simulink model "MCVT_Integration"  (R2020a)
settings: Solver=ode8  StartTime=0.0  StopTime=T_end  FixedStep=dt  RelTol=1e-3  EnableMultiTasking=on
values from neighbouring files (symbols this model uses):
  assumed_power_voltage = 1.25e2    (= 125)    [MCVT_Input_File.m]    (also in MCVT_Run_QuickCheck.m)
  battery_power_toggle = 1    [MCVT_Input_File.m]    (also in MCVT_Run_QuickCheck.m)
  battery_trickle_charge_param = 0.1    [MCVT_Input_File.m]    (also in MCVT_Run_QuickCheck.m)
  battery_trickle_leakage_param = 0.01    [MCVT_Input_File.m]    (also in MCVT_Run_QuickCheck.m)
  damage_case_id = 3    [MCVT_Input_File.m]    (also in MCVT_Run_QuickCheck.m = 1)
  dt = 1/fs    (= 0.001)    [MCVT_Run_QuickCheck.m]    (also in MCVT_Sim_Set.m)
  dt_2 = 1/fs_2    (= 0.001)    [MCVT_Run_QuickCheck.m]    (also in MCVT_Sim_Set.m)
  dt_3 = 1/fs_3    (= 0.001)    [MCVT_Run_QuickCheck.m]    (also in MCVT_Sim_Set.m)
  dt_5 = 1/fs_5    (= 0.005)    [MCVT_Run_QuickCheck.m]    (also in MCVT_Sim_Set.m)
  dt_8 = 1/fs_8    (= 0.05)    [MCVT_Run_QuickCheck.m]    (also in MCVT_Sim_Set.m)
  dt_9 = 1/fs_9    (= 0.001)    [MCVT_Run_QuickCheck.m]    (also in MCVT_Sim_Set.m)
  dust_met_duration = 3    [MCVT_Input_File.m]    (also in MCVT_Run_QuickCheck.m = 2)
  ECLSS_indoor_set_temperature = 298.15    [MCVT_Input_File.m]    (also in MCVT_Run_QuickCheck.m)
  energy_store_max_o = 150    [MCVT_Input_File.m]    (also in MCVT_Run_QuickCheck.m)
  energy_store_o = 120    [MCVT_Input_File.m]    (also in MCVT_Run_QuickCheck.m)
  fs = 1000    [MCVT_Run_QuickCheck.m]    (also in MCVT_Sim_Set.m)
  gain_charge_param = 1    [MCVT_Input_File.m]    (also in MCVT_Run_QuickCheck.m)
  ground_temperature = 373    [MCVT_Habitat_Design_Input_File.m]    (also in MCVT_Run_QuickCheck.m)
  I_max_charge = 800    [MCVT_Input_File.m]    (also in MCVT_Run_QuickCheck.m)
  I_max_discharge = 800    [MCVT_Input_File.m]    (also in MCVT_Run_QuickCheck.m)
  initial_pressure = 1e5    (= 100000)    [MCVT_Input_File.m]    (also in MCVT_Run_QuickCheck.m)
  initial_temperature = 295    [MCVT_Input_File.m]    (also in MCVT_Run_QuickCheck.m)
  interior_leak_rate = 0.00415    [MCVT_Input_File.m]    (also in MCVT_Run_QuickCheck.m)
  launch_dust_accum_start_time = 10    [MCVT_Input_File.m]    (also in MCVT_Run_QuickCheck.m = 2)
  launch_dust_accum_stop_time = 12    [MCVT_Input_File.m]    (also in MCVT_Run_QuickCheck.m = 3)
  meteorite_impact_time = 2    [MCVT_Input_File.m]    (also in MCVT_Run_QuickCheck.m = 1)
  nuclear_power_toggle = 1    [MCVT_Input_File.m]    (also in MCVT_Run_QuickCheck.m)
  print_interval = 30    [MCVT_Input_File.m]
  SOC_lower_thres = 0.5    [MCVT_Input_File.m]    (also in MCVT_Run_QuickCheck.m)
  SOC_upper_thres = 0.9    [MCVT_Input_File.m]    (also in MCVT_Run_QuickCheck.m)
  solar_power_toggle = 1    [MCVT_Input_File.m]    (also in MCVT_Run_QuickCheck.m)
  space_temperature = 4    [MCVT_Habitat_Design_Input_File.m]    (also in MCVT_Run_QuickCheck.m)
  Start_angle = 90    [MCVT_Input_File.m]    (also in MCVT_Run_QuickCheck.m)
undefined symbols (not defined in the file or in its folder): A_flow_restrictor, ACTIVITY_LOOKUP_TABLE, air, Aref, Bmap, Bmap2, Bmap3, comms, DiscIntNeverNeededParam, DmgSPL, dust_parameters, ECLSS_dust_damage_repair_time, ECLSS_heater_capacity, ECLSS_IPS_restrictor, ECLSS_IPS_setpointPressure, ECLSS_paint_damage_repair_time, ECLSS_radiatorLoop_discreteNumber, ECLSS_Vst, emptyActivity, expected_heat_add, FI, habitat, HIEM2SMM, HIEM2STM, HIEMDamageElements, impact_numbering, initial_SPLT_damage_index, input_air, input_Cond, input_CP, input_discretization, input_EV, input_Evap, input_radiatorLoop, K_ECLSS_dust_single, K_ECLSS_paint_single, K_Structure_damage_single, Kref, kW_to_W, meteoroidDustDep, mFRF, ndelays, nominalDustDep, noverlap, nuclear_toggle_gain, output_limits, panel_paint0, Ps0, reference_temperature, RepairTime_ECLSS_factor, Sensor_Location_SMM, SMM_T0, SMM_T1, SMM_T2, SMM_T3, SMM_T4, SMM_T4_0, SMM_T4_1, SMM_T4_2, solar_FDD_K ... (+73)
libraries: neural x350, simulink x39, xpcutilitieslib x7, xpcrtudplib x7, dspstat3 x1
(part 23 of 43)

== system MCVT_Integration/Sys2 - Structural System/Sys2_Structural_System/Fault Detection and Diagnosis (FDD) with Binary Classifer/Pattern Recognition Neural Network/Segment 1/Layer 2/Delays 1 ==
  "p{2}" : Inport  PortDimensions=20
      mask Display: e = 0.06;c1 = [120 230 180]/255;c2 = ([120 230 180]+0)/(256+128);c3 = ([120 230 180]+128)/(256+128);patch([0 1 1 0],[0 0 1 1],c1);patch([0 1 1 1-e 1-e 0+e],[0 0 1 1-e 0+e 0+e],c2);patch([0 0 1 1-e 0+e 0+e],[0 1 1 1-e 1-e 0+e],c3);plot([0 1 1 0 0],[0 0 1 1 0]);
  mux : Mux  Inputs=1
      mask Display: e = 0.06;c1 = [120 150 230]/255;c2 = ([120 150 230]+0)/383;c3 = ([120 150 230]+128)/383;patch([0 1 1 0],[0 0 1 1],c1);patch([0 1 1 1-e 1-e 0+e],[0 0 1 1-e 0+e 0+e],c2);patch([0 0 1 1-e 0+e 0+e],[0 1 1 1-e 1-e 0+e],c3);plot([0 1 1 0 0],[0 0 1 1 0]);disp('Mux')
  "pd{2,1}" : Outport
      mask Display: e = 0.06;c1 = [120 230 180]/255;c2 = ([120 230 180]+0)/(256+128);c3 = ([120 230 180]+128)/(256+128);patch([0 1 1 0],[0 0 1 1],c1);patch([0 1 1 1-e 1-e 0+e],[0 0 1 1-e 0+e 0+e],c2);patch([0 0 1 1-e 0+e 0+e],[0 1 1 1-e 1-e 0+e],c3);plot([0 1 1 0 0],[0 0 1 1 0]);
  wires:
  "p{2}"/1 -> mux/1
  mux/1 -> "pd{2,1}"/1

== system MCVT_Integration/Sys2 - Structural System/Sys2_Structural_System/Fault Detection and Diagnosis (FDD) with Binary Classifer/Pattern Recognition Neural Network/Segment 1/Layer 2/LW{2,1} ==
  "ad{2,1}" : Inport  PortDimensions=20
      mask Display: e = 0.06;c1 = [120 230 180]/255;c2 = ([120 230 180]+0)/(256+128);c3 = ([120 230 180]+128)/(256+128);patch([0 1 1 0],[0 0 1 1],c1);patch([0 1 1 1-e 1-e 0+e],[0 0 1 1-e 0+e 0+e],c2);patch([0 0 1 1-e 0+e 0+e],[0 1 1 1-e 1-e 0+e],c3);plot([0 1 1 0 0],[0 0 1 1 0]);
  "IW{2,1}(1,:)'" : Constant  Value=[-0.25104247195081141885708575500757433474063873291015625;-0.07087667517882868806200491462732315994799137115478515625;-0.50138857313681939320559877160121686756610870361328125;0.470590818650424458358116908129886724054813385009765625;0.10216708641673168111996261586682521738111972808837890625;-0.10534378290408989709003861889868858270347118377685546875;-0.220423474240058869799696594782290048897266387939453125;0.0047890827843588834600296877397340722382068634033203125;-0.172162427952498420236082665724097751080989837646484375;0.02593303469759399015881484729106887243688106536865234375;-0.440084899709885124519104238061117939651012420654296875;0.5753831393220114609476922851172275841236114501953125;0.52466696856238626001101010842830874025821685791015625;-0.027075079370393294320162880239877267740666866302490234375;0.2061943639996793586899315187110914848744869232177734375;-0.65033892131392878610540719819255173206329345703125;-0.54391815389803876978902508199098519980907440185546875;-0.136603011825610132223829396025394089519977569580078125;-0.4909008044092624256649060043855570256710052490234375;-0.43293585150828750585105808568187057971954345703125]
      mask Display: e = 0.06;c1 = [120 150 230]/255;c2 = ([120 150 230]+0)/383;c3 = ([120 150 230]+128)/383;patch([0 1 1 0],[0 0 1 1],c1);patch([0 1 1 1-e 1-e 0+e],[0 0 1 1-e 0+e 0+e],c2);patch([0 0 1 1-e 0+e 0+e],[0 1 1 1-e 1-e 0+e],c3);plot([0 1 1 0 0],[0 0 1 1 0]);disp('weights');
  "IW{2,1}(10,:)'" : Constant  Value=[-0.0014451714761772004036455285813644877634942531585693359375;0.04608926107551207518309155375391128472983837127685546875;-0.11821960628676408955328724914579652249813079833984375;-0.682229667481613955004604576970450580120086669921875;-0.0159400991711729327071633832701991195790469646453857421875;-0.86523788555619007833996647605090402066707611083984375;-0.003532397103530628135192870331593439914286136627197265625;-0.6176597636085656706228519396972842514514923095703125;0.49329025270850446194259575349860824644565582275390625;-0.5412568517979365889658538435469381511211395263671875;0.473563214439475854700134505037567578256130218505859375;-0.1767114189148812075647043684512027539312839508056640625;-0.399614154169822433093628433198318816721439361572265625;0.07107937000623387735487312966142781078815460205078125;-0.345436571249109036774171954675693996250629425048828125;-0.51026032312097269194595128283253870904445648193359375;0.4013803605705799082414841905119828879833221435546875;0.0283768978295916400600429341238850611262023448944091796875;0.384419257535470293429824550912599079310894012451171875;0.156445817512429863338496716096415184438228607177734375]
      mask Display: e = 0.06;c1 = [120 150 230]/255;c2 = ([120 150 230]+0)/383;c3 = ([120 150 230]+128)/383;patch([0 1 1 0],[0 0 1 1],c1);patch([0 1 1 1-e 1-e 0+e],[0 0 1 1-e 0+e 0+e],c2);patch([0 0 1 1-e 0+e 0+e],[0 1 1 1-e 1-e 0+e],c3);plot([0 1 1 0 0],[0 0 1 1 0]);disp('weights');
  "IW{2,1}(11,:)'" : Constant  Value=[0.42081956059319758356451757208560593426227569580078125;-0.022623835269686531912469718008651398122310638427734375;-0.1912529964857672959421819314229651354253292083740234375;0.411268215249822599588469529408030211925506591796875;-0.03376416723225823812715162830500048585236072540283203125;0.028509914893441935801465803024257184006273746490478515625;0.493459475451891893538203248681384138762950897216796875;-0.2255309221203130876087783462935476563870906829833984375;-0.50410452447975073742014728850335814058780670166015625;0.187049216999234102498661513891420327126979827880859375;0.032548129774071281328229332530099782161414623260498046875;-0.1551786688610629905138438289213809184730052947998046875;0.513329365290914996222682020743377506732940673828125;0.55303888864058825536318408921943046152591705322265625;0.45722816612853822704209960647858679294586181640625;-0.0831223966265599301106448137943516485393047332763671875;-0.35699709311150495949505057069472968578338623046875;-0.50985596418355594305893419004860334098339080810546875;-0.488125509118289946375313093085424043238162994384765625;-0.55527675340083815225256103076389990746974945068359375]
      mask Display: e = 0.06;c1 = [120 150 230]/255;c2 = ([120 150 230]+0)/383;c3 = ([120 150 230]+128)/383;patch([0 1 1 0],[0 0 1 1],c1);patch([0 1 1 1-e 1-e 0+e],[0 0 1 1-e 0+e 0+e],c2);patch([0 0 1 1-e 0+e 0+e],[0 1 1 1-e 1-e 0+e],c3);plot([0 1 1 0 0],[0 0 1 1 0]);disp('weights');
  "IW{2,1}(12,:)'" : Constant  Value=[0.236430354655128016627685383355128578841686248779296875;0.321247634226019596059842342583579011261463165283203125;-0.381619419658791370064676584661356173455715179443359375;-0.5603837391530195333189112716354429721832275390625;-0.34218091312457066255348081540432758629322052001953125;0.250109754436278475253629949293099343776702880859375;0.38122207807599906015383339763502590358257293701171875;-0.11789201686204854591100144034498953260481357574462890625;-0.338756734050894514620466679843957535922527313232421875;0.412391661445961343890331818329286761581897735595703125;0.414075134801581867804998182691633701324462890625;0.038104094557145896937644380386700504459440708160400390625;0.0302419039804045948482968952930605155415832996368408203125;0.4887310275038261320190713377087377011775970458984375;0.1411505209161965368469537907003541477024555206298828125;-0.6258221129402887772386065989849157631397247314453125;0.467298676415856484300803685982828028500080108642578125;-0.42901209579906207647326255028019659221172332763671875;-0.240050243971256904718103442064602859318256378173828125;-0.285545664296158741013442750045214779675006866455078125]
      mask Display: e = 0.06;c1 = [120 150 230]/255;c2 = ([120 150 230]+0)/383;c3 = ([120 150 230]+128)/383;patch([0 1 1 0],[0 0 1 1],c1);patch([0 1 1 1-e 1-e 0+e],[0 0 1 1-e 0+e 0+e],c2);patch([0 0 1 1-e 0+e 0+e],[0 1 1 1-e 1-e 0+e],c3);plot([0 1 1 0 0],[0 0 1 1 0]);disp('weights');
  "IW{2,1}(13,:)'" : Constant  Value=[-0.70188226939873032961259013973176479339599609375;-0.052550375883878598648468738474548445083200931549072265625;-0.2149029163891983673639884955264278687536716461181640625;0.4523966484412869082376573715009726583957672119140625;0.447030058856958290025573887760401703417301177978515625;0.91880597605265312832756308125681243836879730224609375;0.332584103805542985465848460080451332032680511474609375;0.474859162877379503697738982737064361572265625;-0.78858829848239386794972460847930051386356353759765625;-0.250963571223336401327941302952240221202373504638671875;-0.09702683642612723591280854407159495167434215545654296875;0.2178231302834403304569121928579988889396190643310546875;-0.0090886058850562327637856441242547589354217052459716796875;-0.053394177694803111877686063735382049344480037689208984375;-0.26261695092550108032725120210670866072177886962890625;0.5229767299097236588067971752025187015533447265625;0.433527928048706379104970665139262564480304718017578125;0.6401460177374802551497623426257632672786712646484375;-0.32168396407409549642153478998807258903980255126953125;0.552601658021480535154523749952204525470733642578125]
      mask Display: e = 0.06;c1 = [120 150 230]/255;c2 = ([120 150 230]+0)/383;c3 = ([120 150 230]+128)/383;patch([0 1 1 0],[0 0 1 1],c1);patch([0 1 1 1-e 1-e 0+e],[0 0 1 1-e 0+e 0+e],c2);patch([0 0 1 1-e 0+e 0+e],[0 1 1 1-e 1-e 0+e],c3);plot([0 1 1 0 0],[0 0 1 1 0]);disp('weights');
  "IW{2,1}(14,:)'" : Constant  Value=[0.55502051895556980820600756487692706286907196044921875;0.322359101059607577877130779597791843116283416748046875;0.419005824050040553974127988112741149961948394775390625;0.007681609285077460602675358813939965330064296722412109375;-0.2410459656664609429110868177303927950561046600341796875;-0.221039781054034156770882191267446614801883697509765625;0.54603930521161980760069809548440389335155487060546875;0.067418959358650554225533824137528426945209503173828125;-0.0323460782665744017716491498504183255136013031005859375;-0.1856337082362842227922783422400243580341339111328125;-0.53405205180679649057395863565034233033657073974609375;0.343953276170277655321427801027311943471431732177734375;-0.16456428638465825375902795713045634329319000244140625;0.393861106754853473876920588736538775265216827392578125;-0.4502608918815782512723444597213529050350189208984375;-0.288216798376219440758205792008084245026111602783203125;0.586902267887376094535056836321018636226654052734375;-0.4647318227858097117177749169059097766876220703125;0.346225802719296649190283687858027406036853790283203125;-0.314419441092286866545890688939834944903850555419921875]
      mask Display: e = 0.06;c1 = [120 150 230]/255;c2 = ([120 150 230]+0)/383;c3 = ([120 150 230]+128)/383;patch([0 1 1 0],[0 0 1 1],c1);patch([0 1 1 1-e 1-e 0+e],[0 0 1 1-e 0+e 0+e],c2);patch([0 0 1 1-e 0+e 0+e],[0 1 1 1-e 1-e 0+e],c3);plot([0 1 1 0 0],[0 0 1 1 0]);disp('weights');
  "IW{2,1}(15,:)'" : Constant  Value=[0.261592048245355812685630780833889730274677276611328125;0.258266746050000051138084700141916982829570770263671875;0.456026828704329367791814320298726670444011688232421875;-0.349416013764789479267847127630375325679779052734375;-0.098985831401622481617863513747579418122768402099609375;-0.87058940329144596237398445737198926508426666259765625;0.7585799820000251880713904029107652604579925537109375;-0.366665360098892223472688556284992955625057220458984375;-0.03618561871887283898363563139355392195284366607666015625;-0.0316960271671848925478798264521174132823944091796875;-0.193254430493304873994730996855651028454303741455078125;-0.583944534337839993298757690354250371456146240234375;0.035927797116458860815502163177370675839483737945556640625;0.294865870939098773106934459065087139606475830078125;-0.0291670979891374033965423251402171445079147815704345703125;0.1935450573362349768746781819572788663208484649658203125;-0.326645720989916898968630221133935265243053436279296875;0.6172263366396790740253663898329250514507293701171875;0.0923742803669448109271655766860931180417537689208984375;-0.07597108288948241361371316315853619016706943511962890625]
      mask Display: e = 0.06;c1 = [120 150 230]/255;c2 = ([120 150 230]+0)/383;c3 = ([120 150 230]+128)/383;patch([0 1 1 0],[0 0 1 1],c1);patch([0 1 1 1-e 1-e 0+e],[0 0 1 1-e 0+e 0+e],c2);patch([0 0 1 1-e 0+e 0+e],[0 1 1 1-e 1-e 0+e],c3);plot([0 1 1 0 0],[0 0 1 1 0]);disp('weights');
  "IW{2,1}(16,:)'" : Constant  Value=[0.49985910112507070390819308158825151622295379638671875;0.262577331451962603381389271817170083522796630859375;0.5669173925971240368681947074946947395801544189453125;-0.60516948282403948411456440226174890995025634765625;-0.1558094289289476963578096047058352269232273101806640625;-0.364683372166025698835056800817255862057209014892578125;0.1420052725001659243986296132788993418216705322265625;0.2141064165498478832905249191753682680428028106689453125;0.6483373794802871348252892857999540865421295166015625;-0.2286739974918611528753586981110856868326663970947265625;-0.3719114816497282038909588663955219089984893798828125;0.334556033774955519088933897364768199622631072998046875;-0.00626378324010478694516823594540255726315081119537353515625;-0.3354810610521266056593958637677133083343505859375;0.384352614846133755488466476890607737004756927490234375;0.00525433332858733607995116443589722621254622936248779296875;0.48238166565563778132030847700661979615688323974609375;0.36215002673653262643682637644815258681774139404296875;0.275033987099670695908315565247903577983379364013671875;0.44176368251344211302011899533681571483612060546875]
      mask Display: e = 0.06;c1 = [120 150 230]/255;c2 = ([120 150 230]+0)/383;c3 = ([120 150 230]+128)/383;patch([0 1 1 0],[0 0 1 1],c1);patch([0 1 1 1-e 1-e 0+e],[0 0 1 1-e 0+e 0+e],c2);patch([0 0 1 1-e 0+e 0+e],[0 1 1 1-e 1-e 0+e],c3);plot([0 1 1 0 0],[0 0 1 1 0]);disp('weights');
  "IW{2,1}(17,:)'" : Constant  Value=[0.035575279491615936777737516649722238071262836456298828125;0.63820684295222973592132120757014490664005279541015625;0.08843938171024399996777987098539597354829311370849609375;0.3337755996214439857538991418550722301006317138671875;0.398638565616782292178044144748128019273281097412109375;0.322075919857517545263903002705774269998073577880859375;0.287977516261365529270932483996148221194744110107421875;0.01627156535218370059237003033558721654117107391357421875;0.74695515831539160966912049843813292682170867919921875;0.012406526388811324468353092242978163994848728179931640625;-0.49938590835118568111994363789563067257404327392578125;0.15703610447656946025318802639958448708057403564453125;-0.50369134077274291261261396357440389692783355712890625;-0.01899445375930586266122190863825380802154541015625;-0.59130984202492431478503931430168449878692626953125;-0.1980273527469609273765627222019247710704803466796875;0.46234292470051752133031186531297862529754638671875;0.28962830103311965057599763895268552005290985107421875;0.0683891751535886938118125044638873077929019927978515625;-0.368999265593023206832867799676023423671722412109375]
      mask Display: e = 0.06;c1 = [120 150 230]/255;c2 = ([120 150 230]+0)/383;c3 = ([120 150 230]+128)/383;patch([0 1 1 0],[0 0 1 1],c1);patch([0 1 1 1-e 1-e 0+e],[0 0 1 1-e 0+e 0+e],c2);patch([0 0 1 1-e 0+e 0+e],[0 1 1 1-e 1-e 0+e],c3);plot([0 1 1 0 0],[0 0 1 1 0]);disp('weights');
  "IW{2,1}(18,:)'" : Constant  Value=[-0.43769587860133818413288508963887579739093780517578125;-0.0228674610406213556224042093845127965323626995086669921875;0.4752100307580253701900119267520494759082794189453125;0.369345836129370386213821575438487343490123748779296875;0.48527390657579816402034111888497136533260345458984375;-0.30491696514473243251330814018729142844676971435546875;-0.056559301626488818481863773968143505044281482696533203125;-0.355738915246859133123535912091028876602649688720703125;-0.2378645485846666296492202263834769837558269500732421875;0.1477450253442366412404140874059521593153476715087890625;-0.265286082355863095383341487831785343587398529052734375;0.2125071781071308507637951379365404136478900909423828125;0.439688757603530377959799579912214539945125579833984375;0.376762247854697307491989022310008294880390167236328125;0.08742251540479260840665887144496082328259944915771484375;0.47800828099567393980606766490382142364978790283203125;0.47201292605969158433509846872766502201557159423828125;0.1458082820372927634888782222333247773349285125732421875;-0.56363881171349328180752991102053783833980560302734375;-0.55964867070200607912511259200982749462127685546875]
      mask Display: e = 0.06;c1 = [120 150 230]/255;c2 = ([120 150 230]+0)/383;c3 = ([120 150 230]+128)/383;patch([0 1 1 0],[0 0 1 1],c1);patch([0 1 1 1-e 1-e 0+e],[0 0 1 1-e 0+e 0+e],c2);patch([0 0 1 1-e 0+e 0+e],[0 1 1 1-e 1-e 0+e],c3);plot([0 1 1 0 0],[0 0 1 1 0]);disp('weights');
  "IW{2,1}(19,:)'" : Constant  Value=[-0.10869814468331075174578614905840368010103702545166015625;-0.53172898051534767116521607022150419652462005615234375;0.53895100944922080810073339307564310729503631591796875;0.2275024496827494357997778706703684292733669281005859375;-0.1548414570509061005676443301126710139214992523193359375;-0.2240896089690270487349010863908915780484676361083984375;0.29916250854529169433959623347618617117404937744140625;0.48469371978502540088129535433836281299591064453125;-0.40527413353439023513402617027168162167072296142578125;-0.484701823798124042941282141327974386513233184814453125;0.51942434438598394930153290260932408273220062255859375;0.279298734095186962367307614840683527290821075439453125;-0.112826646341936009942941154804429970681667327880859375;0.3571995548165904921233959612436592578887939453125;0.037177857134936091731969298734838957898318767547607421875;0.0529656769055748821717344299031537957489490509033203125;0.38298504566654989478280413095490075647830963134765625;0.45388750958881851627069181631668470799922943115234375;-0.07093473670653389906437524814464268274605274200439453125;-0.60655617268914630013654232243425212800502777099609375]
      mask Display: e = 0.06;c1 = [120 150 230]/255;c2 = ([120 150 230]+0)/383;c3 = ([120 150 230]+128)/383;patch([0 1 1 0],[0 0 1 1],c1);patch([0 1 1 1-e 1-e 0+e],[0 0 1 1-e 0+e 0+e],c2);patch([0 0 1 1-e 0+e 0+e],[0 1 1 1-e 1-e 0+e],c3);plot([0 1 1 0 0],[0 0 1 1 0]);disp('weights');
  "IW{2,1}(2,:)'" : Constant  Value=[0.68284517360812169162187501569860614836215972900390625;0.4507082552033494504684085768531076610088348388671875;-0.308119246318860062761046947343857027590274810791015625;-0.253261940275691099788701876605045981705188751220703125;0.60249742819716878283742289568181149661540985107421875;-0.2243071842280256189550158296697190962731838226318359375;0.60620213717168647260535863097175024449825286865234375;0.52832748177859889526786218993947841227054595947265625;0.298698990318473522886932869369047693908214569091796875;-0.0619443896486440992443789355093031190335750579833984375;0.5178886582440653807424268961767666041851043701171875;-0.1274639629612403790570596129327896051108837127685546875;0.376069311629317837297747928460012190043926239013671875;0.415509158099792330620658731277217157185077667236328125;-0.022622134259917180132415381876853643916547298431396484375;-0.1764541137998543174347787498845718801021575927734375;0.1255970564076463735769806362441158853471279144287109375;-0.042025390230417236647753043143893592059612274169921875;0.130315760359159871217826776046422310173511505126953125;0.07427740005411358203968319457999314181506633758544921875]
      mask Display: e = 0.06;c1 = [120 150 230]/255;c2 = ([120 150 230]+0)/383;c3 = ([120 150 230]+128)/383;patch([0 1 1 0],[0 0 1 1],c1);patch([0 1 1 1-e 1-e 0+e],[0 0 1 1-e 0+e 0+e],c2);patch([0 0 1 1-e 0+e 0+e],[0 1 1 1-e 1-e 0+e],c3);plot([0 1 1 0 0],[0 0 1 1 0]);disp('weights');
  "IW{2,1}(20,:)'" : Constant  Value=[0.5684224215114472844589954547700472176074981689453125;0.02120751629153221073931234741394291631877422332763671875;-0.410890208700491255111586497150710783898830413818359375;-0.6306528958771349113732185287517495453357696533203125;-0.219135814485515723060160553359310142695903778076171875;0.2617368508397837079115788583294488489627838134765625;0.009453451597234270586955773296722327359020709991455078125;-0.400816584355880911605396477170870639383792877197265625;0.2200862036068371441199786886500078253448009490966796875;-0.52442764832872279523456882088794372975826263427734375;-0.291627152734451555726735705320606939494609832763671875;0.50779143792216585584498034222633577883243560791015625;-0.1666208401461551635858171493964619003236293792724609375;-0.316343312949595889538301207721815444529056549072265625;-0.49538740896291166837528407995705492794513702392578125;-0.250749733633071680838355632658931426703929901123046875;0.408804246685954442153132504245149902999401092529296875;0.09859252431993337228988139031571336090564727783203125;-0.430159422676064495671965914880274794995784759521484375;0.21240382235424737356055402415222488343715667724609375]
      mask Display: e = 0.06;c1 = [120 150 230]/255;c2 = ([120 150 230]+0)/383;c3 = ([120 150 230]+128)/383;patch([0 1 1 0],[0 0 1 1],c1);patch([0 1 1 1-e 1-e 0+e],[0 0 1 1-e 0+e 0+e],c2);patch([0 0 1 1-e 0+e 0+e],[0 1 1 1-e 1-e 0+e],c3);plot([0 1 1 0 0],[0 0 1 1 0]);disp('weights');
  "IW{2,1}(3,:)'" : Constant  Value=[-0.0307593710585965553205856082286118180491030216217041015625;0.0002436129782347998436187186399592974339611828327178955078125;-0.65745819012910755585465949479839764535427093505859375;-0.61642033559575970524946342266048304736614227294921875;0.259997076974169682994642016637953929603099822998046875;0.14489016174955404725466223680996336042881011962890625;0.0752173185318246451114276851512840948998928070068359375;0.384292226304665140990124427844421006739139556884765625;0.4963587909078246429572800479945726692676544189453125;-0.022099140656966810569716841428089537657797336578369140625;-0.146712712506011533353245113175944425165653228759765625;-0.69540561353011032252879886073060333728790283203125;0.31893978699535063814352042754762805998325347900390625;0.1923372214010869163391959091313765384256839752197265625;0.002488720316779761097814027692720628692768514156341552734375;-0.1974956487883900158042393968571559526026248931884765625;0.032372985675372979719188748504166142083704471588134765625;0.585689470896078479000834704493172466754913330078125;0.478535161104326645098439030334702692925930023193359375;0.272337877717785004083594913026900030672550201416015625]
      mask Display: e = 0.06;c1 = [120 150 230]/255;c2 = ([120 150 230]+0)/383;c3 = ([120 150 230]+128)/383;patch([0 1 1 0],[0 0 1 1],c1);patch([0 1 1 1-e 1-e 0+e],[0 0 1 1-e 0+e 0+e],c2);patch([0 0 1 1-e 0+e 0+e],[0 1 1 1-e 1-e 0+e],c3);plot([0 1 1 0 0],[0 0 1 1 0]);disp('weights');
  "IW{2,1}(4,:)'" : Constant  Value=[-0.556383847551235266593039341387338936328887939453125;0.436802634233961872833873485433286987245082855224609375;0.250350658367628875478061445392086170613765716552734375;-0.27606006633948199091577180297463200986385345458984375;-0.17135978230882586981209669829695485532283782958984375;-0.5104262844452847058818178993533365428447723388671875;0.492220519471961159840844857171759940683841705322265625;0.2103449860634294121819465317457797937095165252685546875;-0.340827063330342794333915890092612244188785552978515625;0.343871184210643388023953548326971940696239471435546875;-0.51486307100237638234574433226953260600566864013671875;0.060624214580098860494228318884779582731425762176513671875;0.407749240833047810195211013706284575164318084716796875;0.4072771296855288714056086973869241774082183837890625;0.39344595251153524628762170323170721530914306640625;-0.52064501325928935759890237022773362696170806884765625;0.042791278010919468755002270654586027376353740692138671875;0.166954777178686064598167604344780556857585906982421875;-0.06969975646280113235064845866872929036617279052734375;0.3643746493983297529695164485019631683826446533203125]
      mask Display: e = 0.06;c1 = [120 150 230]/255;c2 = ([120 150 230]+0)/383;c3 = ([120 150 230]+128)/383;patch([0 1 1 0],[0 0 1 1],c1);patch([0 1 1 1-e 1-e 0+e],[0 0 1 1-e 0+e 0+e],c2);patch([0 0 1 1-e 0+e 0+e],[0 1 1 1-e 1-e 0+e],c3);plot([0 1 1 0 0],[0 0 1 1 0]);disp('weights');
  "IW{2,1}(5,:)'" : Constant  Value=[0.51278071939403258028278287383727729320526123046875;-0.157864135066309085875246864816290326416492462158203125;-0.33610279971475709448469615381327457726001739501953125;-0.476228034272532296800051199170411564409732818603515625;0.0279998345650094258851847683899904950521886348724365234375;0.6381682860961703784852261378546245396137237548828125;-0.1770248038459369122055164780249469913542270660400390625;-0.0254083857277098423832484286322141997516155242919921875;-0.335805515312066538147206529174582101404666900634765625;0.150893024040043954858703045829315669834613800048828125;-0.0989510924031857530547284795829909853637218475341796875;-0.1659897359282971052163446756821940653026103973388671875;0.467310016558783514195596353602013550698757171630859375;-0.620936041976615893389634948107413947582244873046875;0.58445640804912268873039238314959220588207244873046875;-0.1783982673008037977968598397637833841145038604736328125;-0.09833095398130632658340033458443940617144107818603515625;-0.1093127748354998651780789487020228989422321319580078125;0.4457196585726015403139399495557881891727447509765625;-0.445292441303903496763183511575334705412387847900390625]
      mask Display: e = 0.06;c1 = [120 150 230]/255;c2 = ([120 150 230]+0)/383;c3 = ([120 150 230]+128)/383;patch([0 1 1 0],[0 0 1 1],c1);patch([0 1 1 1-e 1-e 0+e],[0 0 1 1-e 0+e 0+e],c2);patch([0 0 1 1-e 0+e 0+e],[0 1 1 1-e 1-e 0+e],c3);plot([0 1 1 0 0],[0 0 1 1 0]);disp('weights');
  "IW{2,1}(6,:)'" : Constant  Value=[0.5001189698293833085784854119992814958095550537109375;-0.5417283168060691433964848329196684062480926513671875;0.50550122088397098085721381721668876707553863525390625;0.292662394636604095143894710417953319847583770751953125;-0.1969915968910886105991409067428321577608585357666015625;-0.06351271877972068657935977853412623517215251922607421875;0.2569845301083357735905110530438832938671112060546875;0.1476072782831410468151744908027467317879199981689453125;-0.497328967217120554433762436019605956971645355224609375;-0.0888765468933903068915469702915288507938385009765625;0.480771050374134090166222676998586393892765045166015625;-0.4234169452732452487708769695018418133258819580078125;0.0425999781010166500561098246180335991084575653076171875;-0.2248736843185309697279450347195961512625217437744140625;0.11672205597611089167298104030123795382678508758544921875;0.25913239617522709767882815867778845131397247314453125;0.4215815832394931295112883162801153957843780517578125;-0.59464420050445132570615669465041719377040863037109375;0.492344508866812968772563863240065984427928924560546875;0.188415853182926529285623473697341978549957275390625]
      mask Display: e = 0.06;c1 = [120 150 230]/255;c2 = ([120 150 230]+0)/383;c3 = ([120 150 230]+128)/383;patch([0 1 1 0],[0 0 1 1],c1);patch([0 1 1 1-e 1-e 0+e],[0 0 1 1-e 0+e 0+e],c2);patch([0 0 1 1-e 0+e 0+e],[0 1 1 1-e 1-e 0+e],c3);plot([0 1 1 0 0],[0 0 1 1 0]);disp('weights');
  "IW{2,1}(7,:)'" : Constant  Value=[0.5830726944670738820519773071282543241977691650390625;0.366310714796492409295325387574848718941211700439453125;-0.087387982566325239641713551463908515870571136474609375;0.0788485845998347090191060715369530953466892242431640625;-0.22309047387350189328714122893870808184146881103515625;0.50205865985555231389980690437369048595428466796875;0.6678643872680243287476287150639109313488006591796875;-0.279909061713649431890615915108355693519115447998046875;-0.305740015510490514838437547950888983905315399169921875;0.43151598882851083249789780893479473888874053955078125;0.372613459927299661700317301438190042972564697265625;-0.39196108657314276957350784869049675762653350830078125;0.387738083307753222950253757517202757298946380615234375;0.350074733619810063434130142923095263540744781494140625;0.06365359891553266924457687991889542900025844573974609375;-0.1568185757360982535146831651218235492706298828125;0.32353860916891352683677496315794996917247772216796875;0.33949657230716623335098347524763084948062896728515625;0.051749458564378812697182041802079766057431697845458984375;-0.434702293295581743137745434069074690341949462890625]
      mask Display: e = 0.06;c1 = [120 150 230]/255;c2 = ([120 150 230]+0)/383;c3 = ([120 150 230]+128)/383;patch([0 1 1 0],[0 0 1 1],c1);patch([0 1 1 1-e 1-e 0+e],[0 0 1 1-e 0+e 0+e],c2);patch([0 0 1 1-e 0+e 0+e],[0 1 1 1-e 1-e 0+e],c3);plot([0 1 1 0 0],[0 0 1 1 0]);disp('weights');
  "IW{2,1}(8,:)'" : Constant  Value=[0.2218031428871449772799451238824985921382904052734375;0.35195397382723470069976201557437889277935028076171875;0.08660919263193035677605990940719493664801120758056640625;0.377323108363323667102662284378311596810817718505859375;0.30708241062934737986012123656109906733036041259765625;-0.269268623610397772605296040637767873704433441162109375;0.20054360811516225293615889313514344394207000732421875;-0.40114689888329990719029183310340158641338348388671875;0.62305131380231026927418724881135858595371246337890625;0.530208114066093028071691151126287877559661865234375;0.1053063225520664281109617377296672202646732330322265625;0.2058249113068855162822501370101235806941986083984375;-0.61504953175007071042301731722545810043811798095703125;0.404218691866809420876194280936033464968204498291015625;-0.460877106638896305579322643097839318215847015380859375;-0.4991443808117228542187149287201464176177978515625;0.10111919054037139809043566174295847304165363311767578125;0.1196547788441794379910021461910218931734561920166015625;0.289995514204500370869510561533388681709766387939453125;-0.0397782438943714289347752810499514453113079071044921875]
      mask Display: e = 0.06;c1 = [120 150 230]/255;c2 = ([120 150 230]+0)/383;c3 = ([120 150 230]+128)/383;patch([0 1 1 0],[0 0 1 1],c1);patch([0 1 1 1-e 1-e 0+e],[0 0 1 1-e 0+e 0+e],c2);patch([0 0 1 1-e 0+e 0+e],[0 1 1 1-e 1-e 0+e],c3);plot([0 1 1 0 0],[0 0 1 1 0]);disp('weights');
  "IW{2,1}(9,:)'" : Constant  Value=[-0.555055779036212282306905763107351958751678466796875;0.494099738717565239998208426186465658247470855712890625;0.1246060390889593694208059559969115070998668670654296875;-0.171940507729196390140913308641756884753704071044921875;0.1932180440188754622088396217804984189569950103759765625;0.3036005532718697441652011548285372555255889892578125;-0.18786107796346762643935335290734656155109405517578125;-0.5159497786189144452606569757335819303989410400390625;-0.42400388421848844711092851866851560771465301513671875;0.432451288145871692858435153539176099002361297607421875;-0.287382162223931258981934888652176596224308013916015625;-0.174222169474380350617792601042310707271099090576171875;-0.268709348839036910039368422076222486793994903564453125;0.041113979428590942399868168877219432033598423004150390625;0.31612551633607299805817092419601976871490478515625;0.624650404556427840674359686090610921382904052734375;0.1580910989950360112654692557043745182454586029052734375;-0.485427924108092379018586370875709690153598785400390625;0.50397423512016137348012989605194889008998870849609375;0.293600644491678497427500360572594217956066131591796875]
      mask Display: e = 0.06;c1 = [120 150 230]/255;c2 = ([120 150 230]+0)/383;c3 = ([120 150 230]+128)/383;patch([0 1 1 0],[0 0 1 1],c1);patch([0 1 1 1-e 1-e 0+e],[0 0 1 1-e 0+e 0+e],c2);patch([0 0 1 1-e 0+e 0+e],[0 1 1 1-e 1-e 0+e],c3);plot([0 1 1 0 0],[0 0 1 1 0]);disp('weights');
  Mux : Mux  Inputs=20
      mask Display: e = 0.06;c1 = [120 150 230]/255;c2 = ([120 150 230]+0)/383;c3 = ([120 150 230]+128)/383;patch([0 1 1 0],[0 0 1 1],c1);patch([0 1 1 1-e 1-e 0+e],[0 0 1 1-e 0+e 0+e],c2);patch([0 0 1 1-e 0+e 0+e],[0 1 1 1-e 1-e 0+e],c3);plot([0 1 1 0 0],[0 0 1 1 0]);disp('Mux');
  dotprod{1..20} (20 alike) : neural/Weight Functions/dotprod
  "lz{2,1}" : Outport
      mask Display: e = 0.06;c1 = [120 230 180]/255;c2 = ([120 230 180]+0)/(256+128);c3 = ([120 230 180]+128)/(256+128);patch([0 1 1 0],[0 0 1 1],c1);patch([0 1 1 1-e 1-e 0+e],[0 0 1 1-e 0+e 0+e],c2);patch([0 0 1 1-e 0+e 0+e],[0 1 1 1-e 1-e 0+e],c3);plot([0 1 1 0 0],[0 0 1 1 0]);
  wires:
  "IW{2,1}(1,:)'"/1 -> dotprod1/1
  "ad{2,1}"/1 -> dotprod1/2, dotprod2/2, dotprod3/2, dotprod4/2, dotprod5/2, dotprod6/2, dotprod7/2, dotprod8/2, dotprod9/2, dotprod10/2, dotprod11/2, dotprod12/2, dotprod13/2, dotprod14/2, dotprod15/2, dotprod16/2, dotprod17/2, dotprod18/2, dotprod19/2, dotprod20/2
  dotprod1/1 -> Mux/1
  "IW{2,1}(2,:)'"/1 -> dotprod2/1
  dotprod2/1 -> Mux/2
  "IW{2,1}(3,:)'"/1 -> dotprod3/1
  dotprod3/1 -> Mux/3
  "IW{2,1}(4,:)'"/1 -> dotprod4/1
  dotprod4/1 -> Mux/4
  "IW{2,1}(5,:)'"/1 -> dotprod5/1
  dotprod5/1 -> Mux/5
  "IW{2,1}(6,:)'"/1 -> dotprod6/1
  dotprod6/1 -> Mux/6
  "IW{2,1}(7,:)'"/1 -> dotprod7/1
  dotprod7/1 -> Mux/7
  "IW{2,1}(8,:)'"/1 -> dotprod8/1
  dotprod8/1 -> Mux/8
  "IW{2,1}(9,:)'"/1 -> dotprod9/1
  dotprod9/1 -> Mux/9
  "IW{2,1}(10,:)'"/1 -> dotprod10/1
  dotprod10/1 -> Mux/10
  "IW{2,1}(11,:)'"/1 -> dotprod11/1
  dotprod11/1 -> Mux/11
  "IW{2,1}(12,:)'"/1 -> dotprod12/1
  dotprod12/1 -> Mux/12
  "IW{2,1}(13,:)'"/1 -> dotprod13/1
  dotprod13/1 -> Mux/13
  "IW{2,1}(14,:)'"/1 -> dotprod14/1
  dotprod14/1 -> Mux/14
  "IW{2,1}(15,:)'"/1 -> dotprod15/1
  dotprod15/1 -> Mux/15
  "IW{2,1}(16,:)'"/1 -> dotprod16/1
  dotprod16/1 -> Mux/16
  "IW{2,1}(17,:)'"/1 -> dotprod17/1
  dotprod17/1 -> Mux/17
  "IW{2,1}(18,:)'"/1 -> dotprod18/1
  dotprod18/1 -> Mux/18
  "IW{2,1}(19,:)'"/1 -> dotprod19/1
  dotprod19/1 -> Mux/19
  "IW{2,1}(20,:)'"/1 -> dotprod20/1
  dotprod20/1 -> Mux/20
  Mux/1 -> "lz{2,1}"/1

== system MCVT_Integration/Sys2 - Structural System/Sys2_Structural_System/Fault Detection and Diagnosis (FDD) with Binary Classifer/Pattern Recognition Neural Network/Segment 1/Layer 3/Delays 1 ==
  "p{3}" : Inport  PortDimensions=20
      mask Display: e = 0.06;c1 = [120 230 180]/255;c2 = ([120 230 180]+0)/(256+128);c3 = ([120 230 180]+128)/(256+128);patch([0 1 1 0],[0 0 1 1],c1);patch([0 1 1 1-e 1-e 0+e],[0 0 1 1-e 0+e 0+e],c2);patch([0 0 1 1-e 0+e 0+e],[0 1 1 1-e 1-e 0+e],c3);plot([0 1 1 0 0],[0 0 1 1 0]);
  mux : Mux  Inputs=1
      mask Display: e = 0.06;c1 = [120 150 230]/255;c2 = ([120 150 230]+0)/383;c3 = ([120 150 230]+128)/383;patch([0 1 1 0],[0 0 1 1],c1);patch([0 1 1 1-e 1-e 0+e],[0 0 1 1-e 0+e 0+e],c2);patch([0 0 1 1-e 0+e 0+e],[0 1 1 1-e 1-e 0+e],c3);plot([0 1 1 0 0],[0 0 1 1 0]);disp('Mux')
  "pd{3,2}" : Outport
      mask Display: e = 0.06;c1 = [120 230 180]/255;c2 = ([120 230 180]+0)/(256+128);c3 = ([120 230 180]+128)/(256+128);patch([0 1 1 0],[0 0 1 1],c1);patch([0 1 1 1-e 1-e 0+e],[0 0 1 1-e 0+e 0+e],c2);patch([0 0 1 1-e 0+e 0+e],[0 1 1 1-e 1-e 0+e],c3);plot([0 1 1 0 0],[0 0 1 1 0]);
  wires:
  "p{3}"/1 -> mux/1
  mux/1 -> "pd{3,2}"/1

== system MCVT_Integration/Sys2 - Structural System/Sys2_Structural_System/Fault Detection and Diagnosis (FDD) with Binary Classifer/Pattern Recognition Neural Network/Segment 1/Layer 3/LW{3,2} ==
  "ad{3,2}" : Inport  PortDimensions=20
      mask Display: e = 0.06;c1 = [120 230 180]/255;c2 = ([120 230 180]+0)/(256+128);c3 = ([120 230 180]+128)/(256+128);patch([0 1 1 0],[0 0 1 1],c1);patch([0 1 1 1-e 1-e 0+e],[0 0 1 1-e 0+e 0+e],c2);patch([0 0 1 1-e 0+e 0+e],[0 1 1 1-e 1-e 0+e],c3);plot([0 1 1 0 0],[0 0 1 1 0]);
  "IW{3,2}(1,:)'" : Constant  Value=[-0.484567585299260528142184512034873478114604949951171875;0.42669992555978486503676094798720441758632659912109375;-0.5796390523624646018419070969684980809688568115234375;0.22421730301437470256331607743049971759319305419921875;0.22424693052733057907488500859471969306468963623046875;-0.310379191286030253760230834814137779176235198974609375;-0.27787612863103483551441286181216128170490264892578125;-0.4851643738068365241389301445451565086841583251953125;0.4489394957408754383010318633751012384891510009765625;-1.0204017050303788582965580644668079912662506103515625;-0.544492546570245483650296591804362833499908447265625;0.286321268730261480062182499750633724033832550048828125;1.4674456148021362000832823468954302370548248291015625;0.1806604486803525533300529559710412286221981048583984375;-0.939646393267568047491522520431317389011383056640625;-0.98877582196743152298523682475206442177295684814453125;-0.9637943132215855968070172821171581745147705078125;-0.51833923472474563443057604672503657639026641845703125;0.300069869229497798723826917921542190015316009521484375;-0.1410151419878878098490559978017699904739856719970703125]
      mask Display: e = 0.06;c1 = [120 150 230]/255;c2 = ([120 150 230]+0)/383;c3 = ([120 150 230]+128)/383;patch([0 1 1 0],[0 0 1 1],c1);patch([0 1 1 1-e 1-e 0+e],[0 0 1 1-e 0+e 0+e],c2);patch([0 0 1 1-e 0+e 0+e],[0 1 1 1-e 1-e 0+e],c3);plot([0 1 1 0 0],[0 0 1 1 0]);disp('weights');
  "IW{3,2}(2,:)'" : Constant  Value=[-0.11950107956568374600880844127459567971527576446533203125;-0.468230761035243803203087509245960973203182220458984375;0.437756421680053187106551604301785118877887725830078125;0.305555068580539102018889252576627768576145172119140625;0.04162873389397074574969082050301949493587017059326171875;-0.85178468790498762519547426563804037868976593017578125;0.4745690868107914628382104638149030506610870361328125;0.53951843255394071885433504576212726533412933349609375;0.023333600254617940794599206810744362883269786834716796875;1.157191253364836303063611921970732510089874267578125;-0.95221053783989850760605122559354640543460845947265625;0.892765439542769545511191608966328203678131103515625;-1.2787832502234390830153643037192523479461669921875;0.04783973502374860853336713262251578271389007568359375;0.53387806030956941327758613624610006809234619140625;-0.12472048188486940800689950492596835829317569732666015625;-0.2143977082940174139213951320925843901932239532470703125;-0.75018097847252052101651997872977517545223236083984375;-0.304525170077943607704895612187101505696773529052734375;0.08754737020083057374986168497343896888196468353271484375]
      mask Display: e = 0.06;c1 = [120 150 230]/255;c2 = ([120 150 230]+0)/383;c3 = ([120 150 230]+128)/383;patch([0 1 1 0],[0 0 1 1],c1);patch([0 1 1 1-e 1-e 0+e],[0 0 1 1-e 0+e 0+e],c2);patch([0 0 1 1-e 0+e 0+e],[0 1 1 1-e 1-e 0+e],c3);plot([0 1 1 0 0],[0 0 1 1 0]);disp('weights');
  Mux : Mux  Inputs=2
      mask Display: e = 0.06;c1 = [120 150 230]/255;c2 = ([120 150 230]+0)/383;c3 = ([120 150 230]+128)/383;patch([0 1 1 0],[0 0 1 1],c1);patch([0 1 1 1-e 1-e 0+e],[0 0 1 1-e 0+e 0+e],c2);patch([0 0 1 1-e 0+e 0+e],[0 1 1 1-e 1-e 0+e],c3);plot([0 1 1 0 0],[0 0 1 1 0]);disp('Mux');
  dotprod1 : neural/Weight Functions/dotprod
  dotprod2 : neural/Weight Functions/dotprod
  "lz{3,2}" : Outport
      mask Display: e = 0.06;c1 = [120 230 180]/255;c2 = ([120 230 180]+0)/(256+128);c3 = ([120 230 180]+128)/(256+128);patch([0 1 1 0],[0 0 1 1],c1);patch([0 1 1 1-e 1-e 0+e],[0 0 1 1-e 0+e 0+e],c2);patch([0 0 1 1-e 0+e 0+e],[0 1 1 1-e 1-e 0+e],c3);plot([0 1 1 0 0],[0 0 1 1 0]);
  wires:
  "IW{3,2}(1,:)'"/1 -> dotprod1/1
  "ad{3,2}"/1 -> dotprod1/2, dotprod2/2
  dotprod1/1 -> Mux/1
  "IW{3,2}(2,:)'"/1 -> dotprod2/1
  dotprod2/1 -> Mux/2
  Mux/1 -> "lz{3,2}"/1

== system MCVT_Integration/Sys2 - Structural System/Sys2_Structural_System/Fault Detection and Diagnosis (FDD) with Binary Classifer/Pattern Recognition Neural Network/Segment 2 (2)/Layer 1/Delays 1 ==
  "p{1}" : Inport  PortDimensions=2304
      mask Display: e = 0.06;c1 = [120 230 180]/255;c2 = ([120 230 180]+0)/(256+128);c3 = ([120 230 180]+128)/(256+128);patch([0 1 1 0],[0 0 1 1],c1);patch([0 1 1 1-e 1-e 0+e],[0 0 1 1-e 0+e 0+e],c2);patch([0 0 1 1-e 0+e 0+e],[0 1 1 1-e 1-e 0+e],c3);plot([0 1 1 0 0],[0 0 1 1 0]);
  mux : Mux  Inputs=1
      mask Display: e = 0.06;c1 = [120 150 230]/255;c2 = ([120 150 230]+0)/383;c3 = ([120 150 230]+128)/383;patch([0 1 1 0],[0 0 1 1],c1);patch([0 1 1 1-e 1-e 0+e],[0 0 1 1-e 0+e 0+e],c2);patch([0 0 1 1-e 0+e 0+e],[0 1 1 1-e 1-e 0+e],c3);plot([0 1 1 0 0],[0 0 1 1 0]);disp('Mux')
  "pd{1,1}" : Outport
      mask Display: e = 0.06;c1 = [120 230 180]/255;c2 = ([120 230 180]+0)/(256+128);c3 = ([120 230 180]+128)/(256+128);patch([0 1 1 0],[0 0 1 1],c1);patch([0 1 1 1-e 1-e 0+e],[0 0 1 1-e 0+e 0+e],c2);patch([0 0 1 1-e 0+e 0+e],[0 1 1 1-e 1-e 0+e],c3);plot([0 1 1 0 0],[0 0 1 1 0]);
  wires:
  "p{1}"/1 -> mux/1
  mux/1 -> "pd{1,1}"/1
